simulink model "HvacActrPosnFilFrnt"  (R2022b)
settings: Solver=FixedStepDiscrete  StartTime=0.0  StopTime=10.0  FixedStep=0.2  RelTol=1e-3
settings Model: Creator=blue.ge  LastModifiedBy=blue.ge
settings DataIOCC: SaveFormat=StructureWithTime
settings OptimizationCC: DefaultParameterBehavior=Inlined  EnhancedBackFolding=on  PassReuseOutputArgsAs=Individual arguments  ZeroExternalMemoryAtStartup=off  ZeroInternalMemoryAtStartup=off  OptimizeBlockOrder=speed  DifferentSizesBufferReuse=on  OptimizationCustomize=off  EfficientTunableParamExpr=on
settings DebuggingCC: ParameterTunabilityLossMsg=error
settings ModelReferenceCC: ModelReferenceNumInstancesAllowed=Single
settings SFSimCC: MATLABDynamicMemAlloc=off
settings RTWCC: Description=AUTOSAR  SystemTargetFile=autosar.tlc  GenCodeOnly=on  TemplateMakefile=ert_default_tmf
settings CodeAppCC: ForceParamTrailComments=on  IgnoreCustomStorageClasses=off  OperatorAnnotations=on  SimulinkDataObjDesc=on  SFDataObjDesc=on  InsertBlockDesc=on
settings STFCustomTargetCC: TargetLangStandard=C99 (ISO)  MultiwordLength=256  ParMdlRefBuildCompliant=on  CompOptLevelCompliant=on  IncludeMdlTerminateFcn=off  CombineOutputUpdateFcns=on  SuppressErrorStatus=on  ERTCustomFileBanners=on  SupportNonFinite=off  SupportComplex=off  SupportContinuousTime=off  SupportNonInlinedSFcns=off  RemoveResetFunc=on  MATLABClassNameForMDSCustomization=Simulink.SoftwareTarget.ERTCustomization  AutosarCompliant=on  UseToolchainInfoCompliant=on  LookupTableObjectStructAxisOrder=2,1,3,4,...  LUTObjectStructOrderEvenSpacing=Size,Table,Breakpoints  SystemTargetFile=autosar.tlc
settings ERTTargetCC: TargetLangStandard=C99 (ISO)  ConcurrentExecutionCompliant=off  IncludeMdlTerminateFcn=off  SuppressErrorStatus=on  SupportNonFinite=off  SupportComplex=off  ModelStepFunctionPrototypeControlCompliant=off  CPPClassGenCompliant=off  AutosarCompliant=on  LookupTableObjectStructAxisOrder=2,1,3,4,...  LUTObjectStructOrderEvenSpacing=Size,Table,Breakpoints  InlinedParameterPlacement=NonHierarchical  ERTCustomFileTemplate=example_file_process.tlc

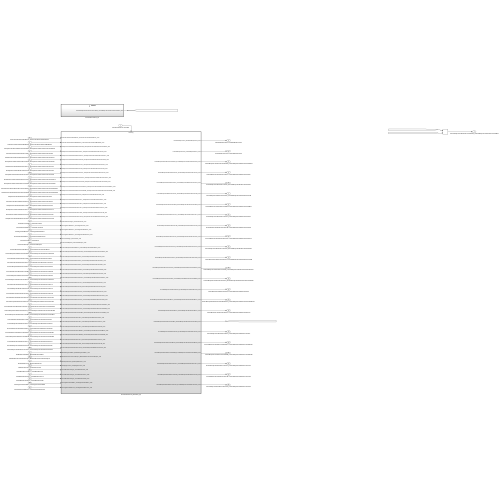
[thumbnail: Simulink's stored preview of the top-level diagram]
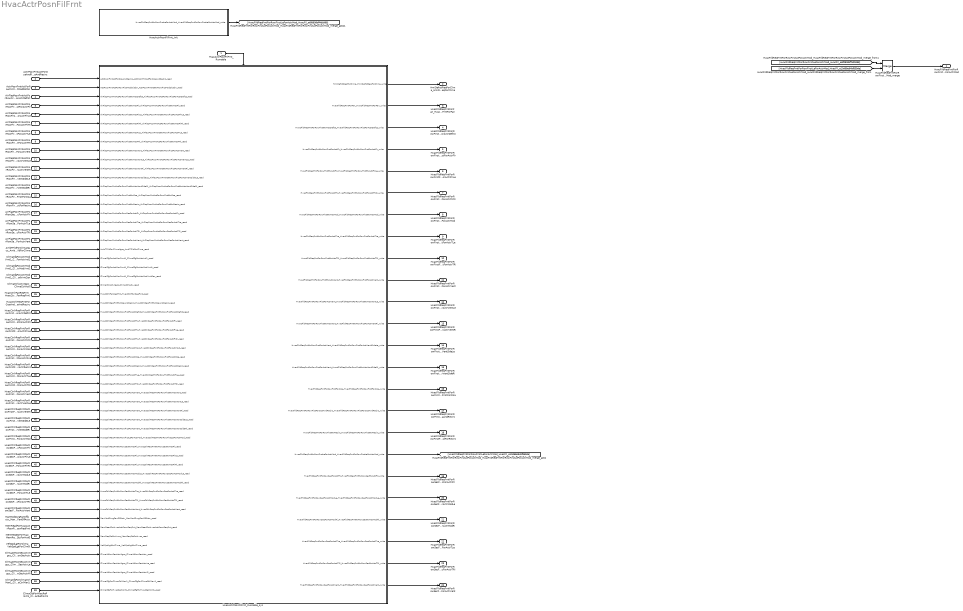
== system HvacActrPosnFilFrnt ==
  HvacActrPosnFilFrnt_Runnable : Inport  SampleTime=0.2  OutputFunctionCall=on
  ActrPosnFrntActForOsaAndRecirc_ActrPosnFrntActForOsaAndRecirc : Inport  Port=2  OutMin=0  OutMax=100  OutDataTypeStr=Perc9  PortDimensions=1
  ActrPosnFrntActForRowFirstModDistbn_ActrPosnFrntActForRowFirstModDistbn : Inport  Port=3  OutMin=0  OutMax=100  OutDataTypeStr=Perc9  PortDimensions=1
  AirFlapPosnFrntActForRowFirstForActrDefrst_AirFlapPosnFrntActForRowFirstForActrDefrst : Inport  Port=4  OutMin=0  OutMax=100  OutDataTypeStr=Perc9  PortDimensions=1
  AirFlapPosnFrntActForRowFirstForActrFlr_AirFlapPosnFrntActForRowFirstForActrFlr : Inport  Port=5  OutMin=0  OutMax=100  OutDataTypeStr=Perc9  PortDimensions=1
  AirFlapPosnFrntActForRowFirstForActrFlrLe_AirFlapPosnFrntActForRowFirstForActrFlrLe : Inport  Port=6  OutMin=0  OutMax=100  OutDataTypeStr=Perc9  PortDimensions=1
  AirFlapPosnFrntActForRowFirstForActrFlrRi_AirFlapPosnFrntActForRowFirstForActrFlrRi : Inport  Port=7  OutMin=0  OutMax=100  OutDataTypeStr=Perc9  PortDimensions=1
  AirFlapPosnFrntActForRowFirstForActrTLe_AirFlapPosnFrntActForRowFirstForActrTLe : Inport  Port=8  OutMin=0  OutMax=100  OutDataTypeStr=Perc9  PortDimensions=1
  AirFlapPosnFrntActForRowFirstForActrTRi_AirFlapPosnFrntActForRowFirstForActrTRi : Inport  Port=9  OutMin=0  OutMax=100  OutDataTypeStr=Perc9  PortDimensions=1
  AirFlapPosnFrntActForRowFirstForActrVent_AirFlapPosnFrntActForRowFirstForActrVent : Inport  Port=10  OutMin=0  OutMax=100  OutDataTypeStr=Perc9  PortDimensions=1
  AirFlapPosnFrntActForRowFirstForActrVentLe_AirFlapPosnFrntActForRowFirstForActrVentLe : Inport  Port=11  OutMin=0  OutMax=100  OutDataTypeStr=Perc9  PortDimensions=1
  AirFlapPosnFrntActForRowFirstForActrVentRi_AirFlapPosnFrntActForRowFirstForActrVentRi : Inport  Port=12  OutMin=0  OutMax=100  OutDataTypeStr=Perc9  PortDimensions=1
  AirFlapPosnFrntActForRowFirstForActrVentSideLe_AirFlapPosnFrntActForRowFirstForActrVentSideLe : Inport  Port=13  OutMin=0  OutMax=100  OutDataTypeStr=Perc9  PortDimensions=1
  AirFlapPosnFrntActForRowFirstForActrVentSideRi_AirFlapPosnFrntActForRowFirstForActrVentSideRi : Inport  Port=14  OutMin=0  OutMax=100  OutDataTypeStr=Perc9  PortDimensions=1
  AirFlapPosnFrntActForRowFirstForOsa_AirFlapPosnFrntActForRowFirstForOsa : Inport  Port=15  OutMin=0  OutMax=100  OutDataTypeStr=Perc9  PortDimensions=1
  AirFlapPosnFrntActForRowFirstForRecirc_AirFlapPosnFrntActForRowFirstForRecirc : Inport  Port=16  OutMin=0  OutMax=100  OutDataTypeStr=Perc9  PortDimensions=1
  AirFlapPosnFrntActForRowSecForActrFlr_AirFlapPosnFrntActForRowSecForActrFlr : Inport  Port=17  OutMin=0  OutMax=100  OutDataTypeStr=Perc9  PortDimensions=1
  AirFlapPosnFrntActForRowSecForActrTLe_AirFlapPosnFrntActForRowSecForActrTLe : Inport  Port=18  OutMin=0  OutMax=100  OutDataTypeStr=Perc9  PortDimensions=1
  AirFlapPosnFrntActForRowSecForActrTRi_AirFlapPosnFrntActForRowSecForActrTRi : Inport  Port=19  OutMin=0  OutMax=100  OutDataTypeStr=Perc9  PortDimensions=1
  AirFlapPosnFrntActForRowSecForActrVent_AirFlapPosnFrntActForRowSecForActrVent : Inport  Port=20  OutMin=0  OutMax=100  OutDataTypeStr=Perc9  PortDimensions=1
  AmbTFildForClimaNgcc_AmbTFildForClima : Inport  Port=21  OutDataTypeStr=T30  PortDimensions=1
  ClimaCfgForActrModVrnt1_ClimaCfgForActrInd1 : Inport  Port=22  OutDataTypeStr=Enum: ClimaCfgForActrInd1  PortDimensions=1
  ClimaCfgForActrModVrnt1_ClimaCfgForActrModVrnt1 : Inport  Port=23  OutDataTypeStr=Enum: ClimaCfgForActrModVrnt1  PortDimensions=1
  ClimaCfgForActrModVrnt1_ClimaCfgForActrModVrntCen : Inport  Port=24  OutDataTypeStr=Enum: ClimaCfgForActrModVrntCen  PortDimensions=1
  ClimaCtrlActvNgcc_ClimaCtrlActv : Inport  Port=25  OutDataTypeStr=Boolean  PortDimensions=1
  HvacCtrlrFanReqFrnt_HvacCtrlrFanReqFrnt : Inport  Port=26  OutDataTypeStr=FanSpd1  PortDimensions=1
  HvacCtrlrReqFrntForOsaAndRecirc_HvacCtrlrReqFrntForOsaAndRecirc : Inport  Port=27  OutDataTypeStr=Perc30  PortDimensions=1
  HvacCtrlrReqFrntForRowFirstForActrDefrst_HvacCtrlrReqFrntForRowFirstForActrDefrst : Inport  Port=28  OutDataTypeStr=Perc30  PortDimensions=1
  HvacCtrlrReqFrntForRowFirstForActrFlr_HvacCtrlrReqFrntForRowFirstForActrFlr : Inport  Port=29  OutDataTypeStr=Perc30  PortDimensions=1
  HvacCtrlrReqFrntForRowFirstForActrFlr_HvacCtrlrReqFrntForRowFirstForActrFlrLe : Inport  Port=30  OutDataTypeStr=Perc30  PortDimensions=1
  HvacCtrlrReqFrntForRowFirstForActrFlr_HvacCtrlrReqFrntForRowFirstForActrFlrRi : Inport  Port=31  OutDataTypeStr=Perc30  PortDimensions=1
  HvacCtrlrReqFrntForRowFirstForActrMod_HvacCtrlrReqFrntForRowFirstForActrMod : Inport  Port=32  OutDataTypeStr=Perc30  PortDimensions=1
  HvacCtrlrReqFrntForRowFirstForActrOsa_HvacCtrlrReqFrntForRowFirstForActrOsa : Inport  Port=33  OutDataTypeStr=Perc30  PortDimensions=1
  HvacCtrlrReqFrntForRowFirstForActrRecirc_HvacCtrlrReqFrntForRowFirstForActrRecirc : Inport  Port=34  OutDataTypeStr=Perc30  PortDimensions=1
  HvacCtrlrReqFrntForRowFirstForActrTLe_HvacCtrlrReqFrntForRowFirstForActrTLe : Inport  Port=35  OutDataTypeStr=Perc30  PortDimensions=1
  HvacCtrlrReqFrntForRowFirstForActrTRi_HvacCtrlrReqFrntForRowFirstForActrTRi : Inport  Port=36  OutDataTypeStr=Perc30  PortDimensions=1
  HvacCtrlrReqFrntForRowFirstForActrVent_HvacCtrlrReqFrntForRowFirstForActrVent : Inport  Port=37  OutDataTypeStr=Perc30  PortDimensions=1
  HvacCtrlrReqFrntForRowFirstForActrVent_HvacCtrlrReqFrntForRowFirstForActrVentLe : Inport  Port=38  OutDataTypeStr=Perc30  PortDimensions=1
  HvacCtrlrReqFrntForRowFirstForActrVent_HvacCtrlrReqFrntForRowFirstForActrVentRi : Inport  Port=39  OutDataTypeStr=Perc30  PortDimensions=1
  HvacCtrlrReqFrntForRowFirstForActrVent_HvacCtrlrReqFrntForRowFirstForActrVentSideLe : Inport  Port=40  OutDataTypeStr=Perc30  PortDimensions=1
  HvacCtrlrReqFrntForRowFirstForActrVent_HvacCtrlrReqFrntForRowFirstForActrVentSideRi : Inport  Port=41  OutDataTypeStr=Perc30  PortDimensions=1
  HvacCtrlrReqFrntForRowFirstLeForActrMod_HvacCtrlrReqFrntForRowFirstLeForActrMod : Inport  Port=42  OutDataTypeStr=Perc30  PortDimensions=1
  HvacCtrlrReqFrntForRowSecForActrFlr_HvacCtrlrReqFrntForRowSecForActrFlr : Inport  Port=43  OutDataTypeStr=Perc30  PortDimensions=1
  HvacCtrlrReqFrntForRowSecForActrFlr_HvacCtrlrReqFrntForRowSecForActrFlrLe : Inport  Port=44  OutDataTypeStr=Perc30  PortDimensions=1
  HvacCtrlrReqFrntForRowSecForActrFlr_HvacCtrlrReqFrntForRowSecForActrFlrRi : Inport  Port=45  OutDataTypeStr=Perc30  PortDimensions=1
  HvacCtrlrReqFrntForRowSecForActrModLe_HvacCtrlrReqFrntForRowSecForActrModLe : Inport  Port=46  OutDataTypeStr=Perc30  PortDimensions=1
  HvacCtrlrReqFrntForRowSecForActrModRi_HvacCtrlrReqFrntForRowSecForActrModRi : Inport  Port=47  OutDataTypeStr=Perc30  PortDimensions=1
  HvacCtrlrReqFrntForRowSecForActrTLe_HvacCtrlrReqFrntForRowSecForActrTLe : Inport  Port=48  OutDataTypeStr=Perc30  PortDimensions=1
  HvacCtrlrReqFrntForRowSecForActrTRi_HvacCtrlrReqFrntForRowSecForActrTRi : Inport  Port=49  OutDataTypeStr=Perc30  PortDimensions=1
  HvacCtrlrReqFrntForRowSecForActrVent_HvacCtrlrReqFrntForRowSecForActrVent : Inport  Port=50  OutDataTypeStr=Perc30  PortDimensions=1
  ManModDurgFanOffActv_ManModDurgFanOffActv : Inport  Port=51  OutDataTypeStr=Boolean  PortDimensions=1
  MemReadForHvacActrPosnReqFrnt_MemReadForHvacActrPosnReqFrnt : Inport  Port=52  OutDataTypeStr=Bus: MemRecForHvacActrPosnReqFrnt  PortDimensions=1  BusOutputAsStruct=on
  MemReqStsForHvac_MemReqStsForHvac : Inport  Port=53  OutDataTypeStr=Enum: MemReqSts  PortDimensions=1
  VehSpdLgtForClima_VehSpdLgtForClima : Inport  Port=54  OutDataTypeStr=Bus: VehSpdLgtForClima  PortDimensions=1  BusOutputAsStruct=on
  ClimaAtRowSecActvNgcc_ClimaAtRowSecActv : Inport  Port=55  OutDataTypeStr=Boolean  PortDimensions=1
  ClimaAtRowSecActvNgcc_ClimaAtRowSecActvLe : Inport  Port=56  OutDataTypeStr=Boolean  PortDimensions=1
  ClimaAtRowSecActvNgcc_ClimaAtRowSecActvRi : Inport  Port=57  OutDataTypeStr=Boolean  PortDimensions=1
  ClimaCfgForClimaCtrlMan1_ClimaCfgForClimaCtrlMan1 : Inport  Port=58  OutDataTypeStr=Boolean  PortDimensions=1
  ClimaCfgForHvacRePrsnt1_ClimaCfgForHvacRePrsnt1 : Inport  Port=59  OutDataTypeStr=Enum: CarCfgForHvacRe  PortDimensions=1
  HvacActrPosnFilFrnt_Init : Subsystem
  HvacActrPosnFilFrnt_Runnable_sys : Subsystem
  HvacFildReqFrntForRowFirstLeForActrMod_HvacFildReqFrntForRowFirstLeForActrMod_merge : Merge
  HvacFildReqFrntForRowFirstLeForActrMod_HvacFildReqFrntForRowFirstLeForActrMod : Outport  OutDataTypeStr=Perc30  PortDimensions=1
  HmiDefrstReqForClima_HmiDefrstReqForClima : Outport  Port=2  OutDataTypeStr=Enum: OnOff1  PortDimensions=1
  HvacFildReqFrntForFan_HvacFildReqFrntForFan : Outport  Port=3  OutDataTypeStr=HvacFanSpd  PortDimensions=1
  HvacFildReqFrntForRowFirstForActrDefrst_HvacFildReqFrntForRowFirstForActrDefrst : Outport  Port=4  OutDataTypeStr=Perc30  PortDimensions=1
  HvacFildReqFrntForRowFirstForActrFlr_HvacFildReqFrntForRowFirstForActrFlr : Outport  Port=5  OutDataTypeStr=Perc30  PortDimensions=1
  HvacFildReqFrntForRowFirstForActrFlr_HvacFildReqFrntForRowFirstForActrFlrLe : Outport  Port=6  OutDataTypeStr=Perc30  PortDimensions=1
  HvacFildReqFrntForRowFirstForActrFlr_HvacFildReqFrntForRowFirstForActrFlrRi : Outport  Port=7  OutDataTypeStr=Perc30  PortDimensions=1
  HvacFildReqFrntForRowFirstForActrMod_HvacFildReqFrntForRowFirstForActrMod : Outport  Port=8  OutDataTypeStr=Perc30  PortDimensions=1
  HvacFildReqFrntForRowFirstForActrTLe_HvacFildReqFrntForRowFirstForActrTLe : Outport  Port=9  OutDataTypeStr=Perc30  PortDimensions=1
  HvacFildReqFrntForRowFirstForActrTRi_HvacFildReqFrntForRowFirstForActrTRi : Outport  Port=10  OutDataTypeStr=Perc30  PortDimensions=1
  HvacFildReqFrntForRowFirstForActrVent_HvacFildReqFrntForRowFirstForActrVent : Outport  Port=11  OutDataTypeStr=Perc30  PortDimensions=1
  HvacFildReqFrntForRowFirstForActrVent_HvacFildReqFrntForRowFirstForActrVentLe : Outport  Port=12  OutDataTypeStr=Perc30  PortDimensions=1
  HvacFildReqFrntForRowFirstForActrVent_HvacFildReqFrntForRowFirstForActrVentRi : Outport  Port=13  OutDataTypeStr=Perc30  PortDimensions=1
  HvacFildReqFrntForRowFirstForActrVent_HvacFildReqFrntForRowFirstForActrVentSideLe : Outport  Port=14  OutDataTypeStr=Perc30  PortDimensions=1
  HvacFildReqFrntForRowFirstForActrVent_HvacFildReqFrntForRowFirstForActrVentSideRi : Outport  Port=15  OutDataTypeStr=Perc30  PortDimensions=1
  HvacFildReqFrntForRowFirstForOsa_HvacFildReqFrntForRowFirstForOsa : Outport  Port=16  OutDataTypeStr=Perc30  PortDimensions=1
  HvacFildReqFrntForRowFirstForOsaAndRecirc_HvacFildReqFrntForRowFirstForOsaAndRecirc : Outport  Port=17  OutDataTypeStr=Perc30  PortDimensions=1
  HvacFildReqFrntForRowFirstForRecirc_HvacFildReqFrntForRowFirstForRecirc : Outport  Port=18  OutDataTypeStr=Perc30  PortDimensions=1
  HvacFildReqFrntForRowSecForActrFlr_HvacFildReqFrntForRowSecForActrFlr : Outport  Port=19  OutDataTypeStr=Perc30  PortDimensions=1
  HvacFildReqFrntForRowSecForActrModLe_HvacFildReqFrntForRowSecForActrModLe : Outport  Port=20  OutDataTypeStr=Perc30  PortDimensions=1
  HvacFildReqFrntForRowSecForActrModRi_HvacFildReqFrntForRowSecForActrModRi : Outport  Port=21  OutDataTypeStr=Perc30  PortDimensions=1
  HvacFildReqFrntForRowSecForActrTLe_HvacFildReqFrntForRowSecForActrTLe : Outport  Port=22  OutDataTypeStr=Perc30  PortDimensions=1
  HvacFildReqFrntForRowSecForActrTRi_HvacFildReqFrntForRowSecForActrTRi : Outport  Port=23  OutDataTypeStr=Perc30  PortDimensions=1
  HvacFildReqFrntForRowSecForActrVent_HvacFildReqFrntForRowSecForActrVent : Outport  Port=24  OutDataTypeStr=Perc30  PortDimensions=1
  wires:
  ActrPosnFrntActForOsaAndRecirc_ActrPosnFrntActForOsaAndRecirc/1 -> HvacActrPosnFilFrnt_Runnable_sys/1(ActrPosnFrntActForOsaAndRecirc_ActrPosnFrntActForOsaAndRecirc_read)
  ActrPosnFrntActForRowFirstModDistbn_ActrPosnFrntActForRowFirstModDistbn/1 -> HvacActrPosnFilFrnt_Runnable_sys/2(ActrPosnFrntActForRowFirstModDistbn_ActrPosnFrntActForRowFirstModDistbn_read)
  AirFlapPosnFrntActForRowFirstForActrDefrst_AirFlapPosnFrntActForRowFirstForActrDefrst/1 -> HvacActrPosnFilFrnt_Runnable_sys/3(AirFlapPosnFrntActForRowFirstForActrDefrst_AirFlapPosnFrntActForRowFirstForActrDefrst_read)
  AirFlapPosnFrntActForRowFirstForActrFlr_AirFlapPosnFrntActForRowFirstForActrFlr/1 -> HvacActrPosnFilFrnt_Runnable_sys/4(AirFlapPosnFrntActForRowFirstForActrFlr_AirFlapPosnFrntActForRowFirstForActrFlr_read)
  AirFlapPosnFrntActForRowFirstForActrFlrLe_AirFlapPosnFrntActForRowFirstForActrFlrLe/1 -> HvacActrPosnFilFrnt_Runnable_sys/5(AirFlapPosnFrntActForRowFirstForActrFlrLe_AirFlapPosnFrntActForRowFirstForActrFlrLe_read)
  AirFlapPosnFrntActForRowFirstForActrFlrRi_AirFlapPosnFrntActForRowFirstForActrFlrRi/1 -> HvacActrPosnFilFrnt_Runnable_sys/6(AirFlapPosnFrntActForRowFirstForActrFlrRi_AirFlapPosnFrntActForRowFirstForActrFlrRi_read)
  AirFlapPosnFrntActForRowFirstForActrTLe_AirFlapPosnFrntActForRowFirstForActrTLe/1 -> HvacActrPosnFilFrnt_Runnable_sys/7(AirFlapPosnFrntActForRowFirstForActrTLe_AirFlapPosnFrntActForRowFirstForActrTLe_read)
  AirFlapPosnFrntActForRowFirstForActrTRi_AirFlapPosnFrntActForRowFirstForActrTRi/1 -> HvacActrPosnFilFrnt_Runnable_sys/8(AirFlapPosnFrntActForRowFirstForActrTRi_AirFlapPosnFrntActForRowFirstForActrTRi_read)
  AirFlapPosnFrntActForRowFirstForActrVent_AirFlapPosnFrntActForRowFirstForActrVent/1 -> HvacActrPosnFilFrnt_Runnable_sys/9(AirFlapPosnFrntActForRowFirstForActrVent_AirFlapPosnFrntActForRowFirstForActrVent_read)
  AirFlapPosnFrntActForRowFirstForActrVentLe_AirFlapPosnFrntActForRowFirstForActrVentLe/1 -> HvacActrPosnFilFrnt_Runnable_sys/10(AirFlapPosnFrntActForRowFirstForActrVentLe_AirFlapPosnFrntActForRowFirstForActrVentLe_read)
  AirFlapPosnFrntActForRowFirstForActrVentRi_AirFlapPosnFrntActForRowFirstForActrVentRi/1 -> HvacActrPosnFilFrnt_Runnable_sys/11(AirFlapPosnFrntActForRowFirstForActrVentRi_AirFlapPosnFrntActForRowFirstForActrVentRi_read)
  AirFlapPosnFrntActForRowFirstForActrVentSideLe_AirFlapPosnFrntActForRowFirstForActrVentSideLe/1 -> HvacActrPosnFilFrnt_Runnable_sys/12(AirFlapPosnFrntActForRowFirstForActrVentSideLe_AirFlapPosnFrntActForRowFirstForActrVentSideLe_read)
  AirFlapPosnFrntActForRowFirstForActrVentSideRi_AirFlapPosnFrntActForRowFirstForActrVentSideRi/1 -> HvacActrPosnFilFrnt_Runnable_sys/13(AirFlapPosnFrntActForRowFirstForActrVentSideRi_AirFlapPosnFrntActForRowFirstForActrVentSideRi_read)
  AirFlapPosnFrntActForRowFirstForOsa_AirFlapPosnFrntActForRowFirstForOsa/1 -> HvacActrPosnFilFrnt_Runnable_sys/14(AirFlapPosnFrntActForRowFirstForOsa_AirFlapPosnFrntActForRowFirstForOsa_read)
  AirFlapPosnFrntActForRowFirstForRecirc_AirFlapPosnFrntActForRowFirstForRecirc/1 -> HvacActrPosnFilFrnt_Runnable_sys/15(AirFlapPosnFrntActForRowFirstForRecirc_AirFlapPosnFrntActForRowFirstForRecirc_read)
  AirFlapPosnFrntActForRowSecForActrFlr_AirFlapPosnFrntActForRowSecForActrFlr/1 -> HvacActrPosnFilFrnt_Runnable_sys/16(AirFlapPosnFrntActForRowSecForActrFlr_AirFlapPosnFrntActForRowSecForActrFlr_read)
  AirFlapPosnFrntActForRowSecForActrTLe_AirFlapPosnFrntActForRowSecForActrTLe/1 -> HvacActrPosnFilFrnt_Runnable_sys/17(AirFlapPosnFrntActForRowSecForActrTLe_AirFlapPosnFrntActForRowSecForActrTLe_read)
  AirFlapPosnFrntActForRowSecForActrTRi_AirFlapPosnFrntActForRowSecForActrTRi/1 -> HvacActrPosnFilFrnt_Runnable_sys/18(AirFlapPosnFrntActForRowSecForActrTRi_AirFlapPosnFrntActForRowSecForActrTRi_read)
  AirFlapPosnFrntActForRowSecForActrVent_AirFlapPosnFrntActForRowSecForActrVent/1 -> HvacActrPosnFilFrnt_Runnable_sys/19(AirFlapPosnFrntActForRowSecForActrVent_AirFlapPosnFrntActForRowSecForActrVent_read)
  AmbTFildForClimaNgcc_AmbTFildForClima/1 -> HvacActrPosnFilFrnt_Runnable_sys/20(AmbTFildForClimaNgcc_AmbTFildForClima_read)
  ClimaCfgForActrModVrnt1_ClimaCfgForActrInd1/1 -> HvacActrPosnFilFrnt_Runnable_sys/21(ClimaCfgForActrModVrnt1_ClimaCfgForActrInd1_read)
  ClimaCfgForActrModVrnt1_ClimaCfgForActrModVrnt1/1 -> HvacActrPosnFilFrnt_Runnable_sys/22(ClimaCfgForActrModVrnt1_ClimaCfgForActrModVrnt1_read)
  ClimaCfgForActrModVrnt1_ClimaCfgForActrModVrntCen/1 -> HvacActrPosnFilFrnt_Runnable_sys/23(ClimaCfgForActrModVrnt1_ClimaCfgForActrModVrntCen_read)
  ClimaCtrlActvNgcc_ClimaCtrlActv/1 -> HvacActrPosnFilFrnt_Runnable_sys/24(ClimaCtrlActvNgcc_ClimaCtrlActv_read)
  HvacCtrlrFanReqFrnt_HvacCtrlrFanReqFrnt/1 -> HvacActrPosnFilFrnt_Runnable_sys/25(HvacCtrlrFanReqFrnt_HvacCtrlrFanReqFrnt_read)
  HvacCtrlrReqFrntForOsaAndRecirc_HvacCtrlrReqFrntForOsaAndRecirc/1 -> HvacActrPosnFilFrnt_Runnable_sys/26(HvacCtrlrReqFrntForOsaAndRecirc_HvacCtrlrReqFrntForOsaAndRecirc_read)
  HvacCtrlrReqFrntForRowFirstForActrDefrst_HvacCtrlrReqFrntForRowFirstForActrDefrst/1 -> HvacActrPosnFilFrnt_Runnable_sys/27(HvacCtrlrReqFrntForRowFirstForActrDefrst_HvacCtrlrReqFrntForRowFirstForActrDefrst_read)
  HvacCtrlrReqFrntForRowFirstForActrFlr_HvacCtrlrReqFrntForRowFirstForActrFlr/1 -> HvacActrPosnFilFrnt_Runnable_sys/28(HvacCtrlrReqFrntForRowFirstForActrFlr_HvacCtrlrReqFrntForRowFirstForActrFlr_read)
  HvacCtrlrReqFrntForRowFirstForActrFlr_HvacCtrlrReqFrntForRowFirstForActrFlrLe/1 -> HvacActrPosnFilFrnt_Runnable_sys/29(HvacCtrlrReqFrntForRowFirstForActrFlr_HvacCtrlrReqFrntForRowFirstForActrFlrLe_read)
  HvacCtrlrReqFrntForRowFirstForActrFlr_HvacCtrlrReqFrntForRowFirstForActrFlrRi/1 -> HvacActrPosnFilFrnt_Runnable_sys/30(HvacCtrlrReqFrntForRowFirstForActrFlr_HvacCtrlrReqFrntForRowFirstForActrFlrRi_read)
  HvacCtrlrReqFrntForRowFirstForActrMod_HvacCtrlrReqFrntForRowFirstForActrMod/1 -> HvacActrPosnFilFrnt_Runnable_sys/31(HvacCtrlrReqFrntForRowFirstForActrMod_HvacCtrlrReqFrntForRowFirstForActrMod_read)
  HvacCtrlrReqFrntForRowFirstForActrOsa_HvacCtrlrReqFrntForRowFirstForActrOsa/1 -> HvacActrPosnFilFrnt_Runnable_sys/32(HvacCtrlrReqFrntForRowFirstForActrOsa_HvacCtrlrReqFrntForRowFirstForActrOsa_read)
  HvacCtrlrReqFrntForRowFirstForActrRecirc_HvacCtrlrReqFrntForRowFirstForActrRecirc/1 -> HvacActrPosnFilFrnt_Runnable_sys/33(HvacCtrlrReqFrntForRowFirstForActrRecirc_HvacCtrlrReqFrntForRowFirstForActrRecirc_read)
  HvacCtrlrReqFrntForRowFirstForActrTLe_HvacCtrlrReqFrntForRowFirstForActrTLe/1 -> HvacActrPosnFilFrnt_Runnable_sys/34(HvacCtrlrReqFrntForRowFirstForActrTLe_HvacCtrlrReqFrntForRowFirstForActrTLe_read)
  HvacCtrlrReqFrntForRowFirstForActrTRi_HvacCtrlrReqFrntForRowFirstForActrTRi/1 -> HvacActrPosnFilFrnt_Runnable_sys/35(HvacCtrlrReqFrntForRowFirstForActrTRi_HvacCtrlrReqFrntForRowFirstForActrTRi_read)
  HvacCtrlrReqFrntForRowFirstForActrVent_HvacCtrlrReqFrntForRowFirstForActrVent/1 -> HvacActrPosnFilFrnt_Runnable_sys/36(HvacCtrlrReqFrntForRowFirstForActrVent_HvacCtrlrReqFrntForRowFirstForActrVent_read)
  HvacCtrlrReqFrntForRowFirstForActrVent_HvacCtrlrReqFrntForRowFirstForActrVentLe/1 -> HvacActrPosnFilFrnt_Runnable_sys/37(HvacCtrlrReqFrntForRowFirstForActrVent_HvacCtrlrReqFrntForRowFirstForActrVentLe_read)
  HvacCtrlrReqFrntForRowFirstForActrVent_HvacCtrlrReqFrntForRowFirstForActrVentRi/1 -> HvacActrPosnFilFrnt_Runnable_sys/38(HvacCtrlrReqFrntForRowFirstForActrVent_HvacCtrlrReqFrntForRowFirstForActrVentRi_read)
  HvacCtrlrReqFrntForRowFirstForActrVent_HvacCtrlrReqFrntForRowFirstForActrVentSideLe/1 -> HvacActrPosnFilFrnt_Runnable_sys/39(HvacCtrlrReqFrntForRowFirstForActrVent_HvacCtrlrReqFrntForRowFirstForActrVentSideLe_read)
  HvacCtrlrReqFrntForRowFirstForActrVent_HvacCtrlrReqFrntForRowFirstForActrVentSideRi/1 -> HvacActrPosnFilFrnt_Runnable_sys/40(HvacCtrlrReqFrntForRowFirstForActrVent_HvacCtrlrReqFrntForRowFirstForActrVentSideRi_read)
  HvacCtrlrReqFrntForRowFirstLeForActrMod_HvacCtrlrReqFrntForRowFirstLeForActrMod/1 -> HvacActrPosnFilFrnt_Runnable_sys/41(HvacCtrlrReqFrntForRowFirstLeForActrMod_HvacCtrlrReqFrntForRowFirstLeForActrMod_read)
  HvacCtrlrReqFrntForRowSecForActrFlr_HvacCtrlrReqFrntForRowSecForActrFlr/1 -> HvacActrPosnFilFrnt_Runnable_sys/42(HvacCtrlrReqFrntForRowSecForActrFlr_HvacCtrlrReqFrntForRowSecForActrFlr_read)
  HvacCtrlrReqFrntForRowSecForActrFlr_HvacCtrlrReqFrntForRowSecForActrFlrLe/1 -> HvacActrPosnFilFrnt_Runnable_sys/43(HvacCtrlrReqFrntForRowSecForActrFlr_HvacCtrlrReqFrntForRowSecForActrFlrLe_read)
  HvacCtrlrReqFrntForRowSecForActrFlr_HvacCtrlrReqFrntForRowSecForActrFlrRi/1 -> HvacActrPosnFilFrnt_Runnable_sys/44(HvacCtrlrReqFrntForRowSecForActrFlr_HvacCtrlrReqFrntForRowSecForActrFlrRi_read)
  HvacCtrlrReqFrntForRowSecForActrModLe_HvacCtrlrReqFrntForRowSecForActrModLe/1 -> HvacActrPosnFilFrnt_Runnable_sys/45(HvacCtrlrReqFrntForRowSecForActrModLe_HvacCtrlrReqFrntForRowSecForActrModLe_read)
  HvacCtrlrReqFrntForRowSecForActrModRi_HvacCtrlrReqFrntForRowSecForActrModRi/1 -> HvacActrPosnFilFrnt_Runnable_sys/46(HvacCtrlrReqFrntForRowSecForActrModRi_HvacCtrlrReqFrntForRowSecForActrModRi_read)
  HvacCtrlrReqFrntForRowSecForActrTLe_HvacCtrlrReqFrntForRowSecForActrTLe/1 -> HvacActrPosnFilFrnt_Runnable_sys/47(HvacCtrlrReqFrntForRowSecForActrTLe_HvacCtrlrReqFrntForRowSecForActrTLe_read)
  HvacCtrlrReqFrntForRowSecForActrTRi_HvacCtrlrReqFrntForRowSecForActrTRi/1 -> HvacActrPosnFilFrnt_Runnable_sys/48(HvacCtrlrReqFrntForRowSecForActrTRi_HvacCtrlrReqFrntForRowSecForActrTRi_read)
  HvacCtrlrReqFrntForRowSecForActrVent_HvacCtrlrReqFrntForRowSecForActrVent/1 -> HvacActrPosnFilFrnt_Runnable_sys/49(HvacCtrlrReqFrntForRowSecForActrVent_HvacCtrlrReqFrntForRowSecForActrVent_read)
  ManModDurgFanOffActv_ManModDurgFanOffActv/1 -> HvacActrPosnFilFrnt_Runnable_sys/50(ManModDurgFanOffActv_ManModDurgFanOffActv_read)
  MemReadForHvacActrPosnReqFrnt_MemReadForHvacActrPosnReqFrnt/1 -> HvacActrPosnFilFrnt_Runnable_sys/51(MemReadForHvacActrPosnReqFrnt_MemReadForHvacActrPosnReqFrnt_read)
  MemReqStsForHvac_MemReqStsForHvac/1 -> HvacActrPosnFilFrnt_Runnable_sys/52(MemReqStsForHvac_MemReqStsForHvac_read)
  VehSpdLgtForClima_VehSpdLgtForClima/1 -> HvacActrPosnFilFrnt_Runnable_sys/53(VehSpdLgtForClima_VehSpdLgtForClima_read)
  ClimaAtRowSecActvNgcc_ClimaAtRowSecActv/1 -> HvacActrPosnFilFrnt_Runnable_sys/54(ClimaAtRowSecActvNgcc_ClimaAtRowSecActv_read)
  ClimaAtRowSecActvNgcc_ClimaAtRowSecActvLe/1 -> HvacActrPosnFilFrnt_Runnable_sys/55(ClimaAtRowSecActvNgcc_ClimaAtRowSecActvLe_read)
  ClimaAtRowSecActvNgcc_ClimaAtRowSecActvRi/1 -> HvacActrPosnFilFrnt_Runnable_sys/56(ClimaAtRowSecActvNgcc_ClimaAtRowSecActvRi_read)
  ClimaCfgForClimaCtrlMan1_ClimaCfgForClimaCtrlMan1/1 -> HvacActrPosnFilFrnt_Runnable_sys/57(ClimaCfgForClimaCtrlMan1_ClimaCfgForClimaCtrlMan1_read)
  ClimaCfgForHvacRePrsnt1_ClimaCfgForHvacRePrsnt1/1 -> HvacActrPosnFilFrnt_Runnable_sys/58(ClimaCfgForHvacRePrsnt1_ClimaCfgForHvacRePrsnt1_read)
  HvacActrPosnFilFrnt_Runnable_sys/1(HmiDefrstReqForClima_HmiDefrstReqForClima_write) -> HmiDefrstReqForClima_HmiDefrstReqForClima/1
  HvacActrPosnFilFrnt_Runnable_sys/2(HvacFildReqFrntForFan_HvacFildReqFrntForFan_write) -> HvacFildReqFrntForFan_HvacFildReqFrntForFan/1
  HvacActrPosnFilFrnt_Runnable_sys/3(HvacFildReqFrntForRowFirstForActrDefrst_HvacFildReqFrntForRowFirstForActrDefrst_write) -> HvacFildReqFrntForRowFirstForActrDefrst_HvacFildReqFrntForRowFirstForActrDefrst/1
  HvacActrPosnFilFrnt_Runnable_sys/4(HvacFildReqFrntForRowFirstForActrFlr_HvacFildReqFrntForRowFirstForActrFlr_write) -> HvacFildReqFrntForRowFirstForActrFlr_HvacFildReqFrntForRowFirstForActrFlr/1
  HvacActrPosnFilFrnt_Runnable_sys/5(HvacFildReqFrntForRowFirstForActrFlr_HvacFildReqFrntForRowFirstForActrFlrLe_write) -> HvacFildReqFrntForRowFirstForActrFlr_HvacFildReqFrntForRowFirstForActrFlrLe/1
  HvacActrPosnFilFrnt_Runnable_sys/6(HvacFildReqFrntForRowFirstForActrFlr_HvacFildReqFrntForRowFirstForActrFlrRi_write) -> HvacFildReqFrntForRowFirstForActrFlr_HvacFildReqFrntForRowFirstForActrFlrRi/1
  HvacActrPosnFilFrnt_Runnable_sys/7(HvacFildReqFrntForRowFirstForActrMod_HvacFildReqFrntForRowFirstForActrMod_write) -> HvacFildReqFrntForRowFirstForActrMod_HvacFildReqFrntForRowFirstForActrMod/1
  HvacActrPosnFilFrnt_Runnable_sys/8(HvacFildReqFrntForRowFirstForActrTLe_HvacFildReqFrntForRowFirstForActrTLe_write) -> HvacFildReqFrntForRowFirstForActrTLe_HvacFildReqFrntForRowFirstForActrTLe/1
  HvacActrPosnFilFrnt_Runnable_sys/9(HvacFildReqFrntForRowFirstForActrTRi_HvacFildReqFrntForRowFirstForActrTRi_write) -> HvacFildReqFrntForRowFirstForActrTRi_HvacFildReqFrntForRowFirstForActrTRi/1
  HvacActrPosnFilFrnt_Runnable_sys/10(HvacFildReqFrntForRowFirstForActrVent_HvacFildReqFrntForRowFirstForActrVent_write) -> HvacFildReqFrntForRowFirstForActrVent_HvacFildReqFrntForRowFirstForActrVent/1
  HvacActrPosnFilFrnt_Runnable_sys/11(HvacFildReqFrntForRowFirstForActrVent_HvacFildReqFrntForRowFirstForActrVentLe_write) -> HvacFildReqFrntForRowFirstForActrVent_HvacFildReqFrntForRowFirstForActrVentLe/1
  HvacActrPosnFilFrnt_Runnable_sys/12(HvacFildReqFrntForRowFirstForActrVent_HvacFildReqFrntForRowFirstForActrVentRi_write) -> HvacFildReqFrntForRowFirstForActrVent_HvacFildReqFrntForRowFirstForActrVentRi/1
  HvacActrPosnFilFrnt_Runnable_sys/13(HvacFildReqFrntForRowFirstForActrVent_HvacFildReqFrntForRowFirstForActrVentSideLe_write) -> HvacFildReqFrntForRowFirstForActrVent_HvacFildReqFrntForRowFirstForActrVentSideLe/1
  HvacActrPosnFilFrnt_Runnable_sys/14(HvacFildReqFrntForRowFirstForActrVent_HvacFildReqFrntForRowFirstForActrVentSideRi_write) -> HvacFildReqFrntForRowFirstForActrVent_HvacFildReqFrntForRowFirstForActrVentSideRi/1
  HvacActrPosnFilFrnt_Runnable_sys/15(HvacFildReqFrntForRowFirstForOsa_HvacFildReqFrntForRowFirstForOsa_write) -> HvacFildReqFrntForRowFirstForOsa_HvacFildReqFrntForRowFirstForOsa/1
  HvacActrPosnFilFrnt_Runnable_sys/16(HvacFildReqFrntForRowFirstForOsaAndRecirc_HvacFildReqFrntForRowFirstForOsaAndRecirc_write) -> HvacFildReqFrntForRowFirstForOsaAndRecirc_HvacFildReqFrntForRowFirstForOsaAndRecirc/1
  HvacActrPosnFilFrnt_Runnable_sys/17(HvacFildReqFrntForRowFirstForRecirc_HvacFildReqFrntForRowFirstForRecirc_write) -> HvacFildReqFrntForRowFirstForRecirc_HvacFildReqFrntForRowFirstForRecirc/1
  HvacFildReqFrntForRowFirstLeForActrMod_HvacFildReqFrntForRowFirstLeForActrMod_merge/1 -> HvacFildReqFrntForRowFirstLeForActrMod_HvacFildReqFrntForRowFirstLeForActrMod/1
  HvacActrPosnFilFrnt_Runnable_sys/19(HvacFildReqFrntForRowSecForActrFlr_HvacFildReqFrntForRowSecForActrFlr_write) -> HvacFildReqFrntForRowSecForActrFlr_HvacFildReqFrntForRowSecForActrFlr/1
  HvacActrPosnFilFrnt_Runnable_sys/20(HvacFildReqFrntForRowSecForActrModLe_HvacFildReqFrntForRowSecForActrModLe_write) -> HvacFildReqFrntForRowSecForActrModLe_HvacFildReqFrntForRowSecForActrModLe/1
  HvacActrPosnFilFrnt_Runnable_sys/21(HvacFildReqFrntForRowSecForActrModRi_HvacFildReqFrntForRowSecForActrModRi_write) -> HvacFildReqFrntForRowSecForActrModRi_HvacFildReqFrntForRowSecForActrModRi/1
  HvacActrPosnFilFrnt_Runnable_sys/22(HvacFildReqFrntForRowSecForActrTLe_HvacFildReqFrntForRowSecForActrTLe_write) -> HvacFildReqFrntForRowSecForActrTLe_HvacFildReqFrntForRowSecForActrTLe/1
  HvacActrPosnFilFrnt_Runnable_sys/23(HvacFildReqFrntForRowSecForActrTRi_HvacFildReqFrntForRowSecForActrTRi_write) -> HvacFildReqFrntForRowSecForActrTRi_HvacFildReqFrntForRowSecForActrTRi/1
  HvacActrPosnFilFrnt_Runnable_sys/24(HvacFildReqFrntForRowSecForActrVent_HvacFildReqFrntForRowSecForActrVent_write) -> HvacFildReqFrntForRowSecForActrVent_HvacFildReqFrntForRowSecForActrVent/1
  HvacActrPosnFilFrnt_Runnable_sys/18(HvacFildReqFrntForRowFirstLeForActrMod_HvacFildReqFrntForRowFirstLeForActrMod_write) -> [HvacFildReqFrntForRowFirstLeForActrMod_HvacFil_e10d68e94bf859de]
  [HvacFildReqFrntForRowFirstLeForActrMod_HvacFil_e10d68e94bf859de] -> HvacFildReqFrntForRowFirstLeForActrMod_HvacFildReqFrntForRowFirstLeForActrMod_merge/2
  HvacActrPosnFilFrnt_Init/1(HvacFildReqFrntForRowFirstLeForActrMod_HvacFildReqFrntForRowFirstLeForActrMod_write) -> [HvacFildReqFrntForRowFirstLeForActrMod_HvacFil_e6fd808bff4db998]
  [HvacFildReqFrntForRowFirstLeForActrMod_HvacFil_e6fd808bff4db998] -> HvacFildReqFrntForRowFirstLeForActrMod_HvacFildReqFrntForRowFirstLeForActrMod_merge/1
  HvacActrPosnFilFrnt_Runnable/1 -> HvacActrPosnFilFrnt_Runnable_sys/Trigger

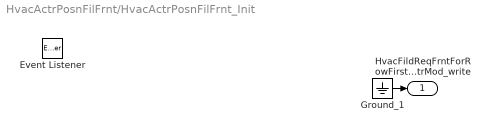
== system HvacActrPosnFilFrnt/HvacActrPosnFilFrnt_Init ==
  Event Listener : EventListener  EventName=reset
  Ground_1 : Ground
  HvacFildReqFrntForRowFirstLeForActrMod_HvacFildReqFrntForRowFirstLeForActrMod_write : Outport
  wires:
  Ground_1/1 -> HvacFildReqFrntForRowFirstLeForActrMod_HvacFildReqFrntForRowFirstLeForActrMod_write/1

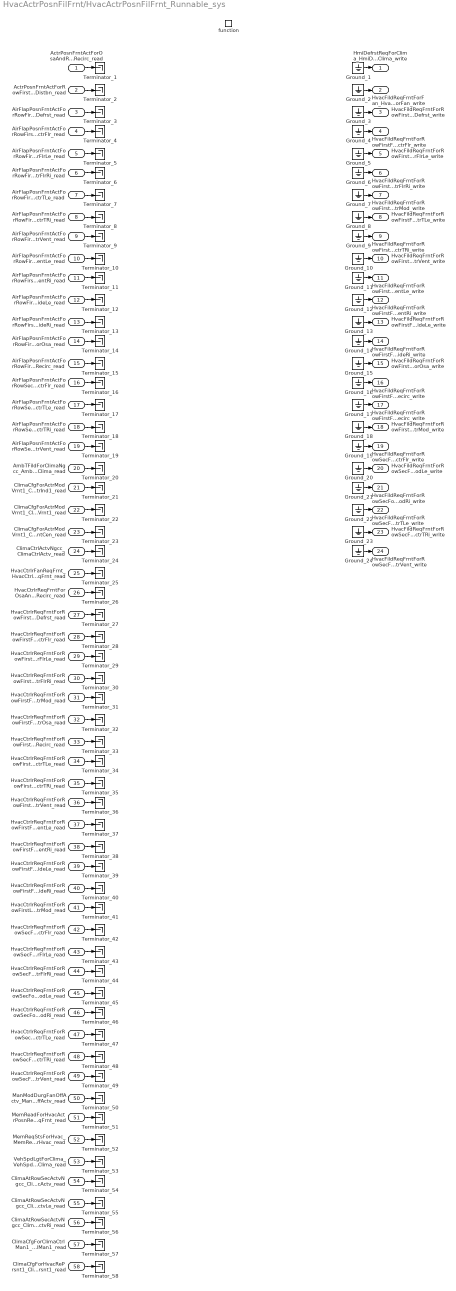
== system HvacActrPosnFilFrnt/HvacActrPosnFilFrnt_Runnable_sys ==
  ActrPosnFrntActForOsaAndRecirc_ActrPosnFrntActForOsaAndRecirc_read : Inport
  ActrPosnFrntActForRowFirstModDistbn_ActrPosnFrntActForRowFirstModDistbn_read : Inport  Port=2
  AirFlapPosnFrntActForRowFirstForActrDefrst_AirFlapPosnFrntActForRowFirstForActrDefrst_read : Inport  Port=3
  AirFlapPosnFrntActForRowFirstForActrFlr_AirFlapPosnFrntActForRowFirstForActrFlr_read : Inport  Port=4
  AirFlapPosnFrntActForRowFirstForActrFlrLe_AirFlapPosnFrntActForRowFirstForActrFlrLe_read : Inport  Port=5
  AirFlapPosnFrntActForRowFirstForActrFlrRi_AirFlapPosnFrntActForRowFirstForActrFlrRi_read : Inport  Port=6
  AirFlapPosnFrntActForRowFirstForActrTLe_AirFlapPosnFrntActForRowFirstForActrTLe_read : Inport  Port=7
  AirFlapPosnFrntActForRowFirstForActrTRi_AirFlapPosnFrntActForRowFirstForActrTRi_read : Inport  Port=8
  AirFlapPosnFrntActForRowFirstForActrVent_AirFlapPosnFrntActForRowFirstForActrVent_read : Inport  Port=9
  AirFlapPosnFrntActForRowFirstForActrVentLe_AirFlapPosnFrntActForRowFirstForActrVentLe_read : Inport  Port=10
  AirFlapPosnFrntActForRowFirstForActrVentRi_AirFlapPosnFrntActForRowFirstForActrVentRi_read : Inport  Port=11
  AirFlapPosnFrntActForRowFirstForActrVentSideLe_AirFlapPosnFrntActForRowFirstForActrVentSideLe_read : Inport  Port=12
  AirFlapPosnFrntActForRowFirstForActrVentSideRi_AirFlapPosnFrntActForRowFirstForActrVentSideRi_read : Inport  Port=13
  AirFlapPosnFrntActForRowFirstForOsa_AirFlapPosnFrntActForRowFirstForOsa_read : Inport  Port=14
  AirFlapPosnFrntActForRowFirstForRecirc_AirFlapPosnFrntActForRowFirstForRecirc_read : Inport  Port=15
  AirFlapPosnFrntActForRowSecForActrFlr_AirFlapPosnFrntActForRowSecForActrFlr_read : Inport  Port=16
  AirFlapPosnFrntActForRowSecForActrTLe_AirFlapPosnFrntActForRowSecForActrTLe_read : Inport  Port=17
  AirFlapPosnFrntActForRowSecForActrTRi_AirFlapPosnFrntActForRowSecForActrTRi_read : Inport  Port=18
  AirFlapPosnFrntActForRowSecForActrVent_AirFlapPosnFrntActForRowSecForActrVent_read : Inport  Port=19
  AmbTFildForClimaNgcc_AmbTFildForClima_read : Inport  Port=20
  ClimaCfgForActrModVrnt1_ClimaCfgForActrInd1_read : Inport  Port=21
  ClimaCfgForActrModVrnt1_ClimaCfgForActrModVrnt1_read : Inport  Port=22
  ClimaCfgForActrModVrnt1_ClimaCfgForActrModVrntCen_read : Inport  Port=23
  ClimaCtrlActvNgcc_ClimaCtrlActv_read : Inport  Port=24
  HvacCtrlrFanReqFrnt_HvacCtrlrFanReqFrnt_read : Inport  Port=25
  HvacCtrlrReqFrntForOsaAndRecirc_HvacCtrlrReqFrntForOsaAndRecirc_read : Inport  Port=26
  HvacCtrlrReqFrntForRowFirstForActrDefrst_HvacCtrlrReqFrntForRowFirstForActrDefrst_read : Inport  Port=27
  HvacCtrlrReqFrntForRowFirstForActrFlr_HvacCtrlrReqFrntForRowFirstForActrFlr_read : Inport  Port=28
  HvacCtrlrReqFrntForRowFirstForActrFlr_HvacCtrlrReqFrntForRowFirstForActrFlrLe_read : Inport  Port=29
  HvacCtrlrReqFrntForRowFirstForActrFlr_HvacCtrlrReqFrntForRowFirstForActrFlrRi_read : Inport  Port=30
  HvacCtrlrReqFrntForRowFirstForActrMod_HvacCtrlrReqFrntForRowFirstForActrMod_read : Inport  Port=31
  HvacCtrlrReqFrntForRowFirstForActrOsa_HvacCtrlrReqFrntForRowFirstForActrOsa_read : Inport  Port=32
  HvacCtrlrReqFrntForRowFirstForActrRecirc_HvacCtrlrReqFrntForRowFirstForActrRecirc_read : Inport  Port=33
  HvacCtrlrReqFrntForRowFirstForActrTLe_HvacCtrlrReqFrntForRowFirstForActrTLe_read : Inport  Port=34
  HvacCtrlrReqFrntForRowFirstForActrTRi_HvacCtrlrReqFrntForRowFirstForActrTRi_read : Inport  Port=35
  HvacCtrlrReqFrntForRowFirstForActrVent_HvacCtrlrReqFrntForRowFirstForActrVent_read : Inport  Port=36
  HvacCtrlrReqFrntForRowFirstForActrVent_HvacCtrlrReqFrntForRowFirstForActrVentLe_read : Inport  Port=37
  HvacCtrlrReqFrntForRowFirstForActrVent_HvacCtrlrReqFrntForRowFirstForActrVentRi_read : Inport  Port=38
  HvacCtrlrReqFrntForRowFirstForActrVent_HvacCtrlrReqFrntForRowFirstForActrVentSideLe_read : Inport  Port=39
  HvacCtrlrReqFrntForRowFirstForActrVent_HvacCtrlrReqFrntForRowFirstForActrVentSideRi_read : Inport  Port=40
  HvacCtrlrReqFrntForRowFirstLeForActrMod_HvacCtrlrReqFrntForRowFirstLeForActrMod_read : Inport  Port=41
  HvacCtrlrReqFrntForRowSecForActrFlr_HvacCtrlrReqFrntForRowSecForActrFlr_read : Inport  Port=42
  HvacCtrlrReqFrntForRowSecForActrFlr_HvacCtrlrReqFrntForRowSecForActrFlrLe_read : Inport  Port=43
  HvacCtrlrReqFrntForRowSecForActrFlr_HvacCtrlrReqFrntForRowSecForActrFlrRi_read : Inport  Port=44
  HvacCtrlrReqFrntForRowSecForActrModLe_HvacCtrlrReqFrntForRowSecForActrModLe_read : Inport  Port=45
  HvacCtrlrReqFrntForRowSecForActrModRi_HvacCtrlrReqFrntForRowSecForActrModRi_read : Inport  Port=46
  HvacCtrlrReqFrntForRowSecForActrTLe_HvacCtrlrReqFrntForRowSecForActrTLe_read : Inport  Port=47
  HvacCtrlrReqFrntForRowSecForActrTRi_HvacCtrlrReqFrntForRowSecForActrTRi_read : Inport  Port=48
  HvacCtrlrReqFrntForRowSecForActrVent_HvacCtrlrReqFrntForRowSecForActrVent_read : Inport  Port=49
  ManModDurgFanOffActv_ManModDurgFanOffActv_read : Inport  Port=50
  MemReadForHvacActrPosnReqFrnt_MemReadForHvacActrPosnReqFrnt_read : Inport  Port=51
  MemReqStsForHvac_MemReqStsForHvac_read : Inport  Port=52
  VehSpdLgtForClima_VehSpdLgtForClima_read : Inport  Port=53
  ClimaAtRowSecActvNgcc_ClimaAtRowSecActv_read : Inport  Port=54
  ClimaAtRowSecActvNgcc_ClimaAtRowSecActvLe_read : Inport  Port=55
  ClimaAtRowSecActvNgcc_ClimaAtRowSecActvRi_read : Inport  Port=56
  ClimaCfgForClimaCtrlMan1_ClimaCfgForClimaCtrlMan1_read : Inport  Port=57
  ClimaCfgForHvacRePrsnt1_ClimaCfgForHvacRePrsnt1_read : Inport  Port=58
  function : TriggerPort  TriggerType=function-call  VariantControl=(inherit)  SampleTimeType=periodic  SampleTime=-1
  Ground_{1..24} (24 alike) : Ground
  Terminator_{1..58} (58 alike) : Terminator
  HmiDefrstReqForClima_HmiDefrstReqForClima_write : Outport
      settings: EnsureOutportIsVirtual=on
  HvacFildReqFrntForFan_HvacFildReqFrntForFan_write : Outport  Port=2
      settings: EnsureOutportIsVirtual=on
  HvacFildReqFrntForRowFirstForActrDefrst_HvacFildReqFrntForRowFirstForActrDefrst_write : Outport  Port=3
      settings: EnsureOutportIsVirtual=on
  HvacFildReqFrntForRowFirstForActrFlr_HvacFildReqFrntForRowFirstForActrFlr_write : Outport  Port=4
      settings: EnsureOutportIsVirtual=on
  HvacFildReqFrntForRowFirstForActrFlr_HvacFildReqFrntForRowFirstForActrFlrLe_write : Outport  Port=5
      settings: EnsureOutportIsVirtual=on
  HvacFildReqFrntForRowFirstForActrFlr_HvacFildReqFrntForRowFirstForActrFlrRi_write : Outport  Port=6
      settings: EnsureOutportIsVirtual=on
  HvacFildReqFrntForRowFirstForActrMod_HvacFildReqFrntForRowFirstForActrMod_write : Outport  Port=7
      settings: EnsureOutportIsVirtual=on
  HvacFildReqFrntForRowFirstForActrTLe_HvacFildReqFrntForRowFirstForActrTLe_write : Outport  Port=8
      settings: EnsureOutportIsVirtual=on
  HvacFildReqFrntForRowFirstForActrTRi_HvacFildReqFrntForRowFirstForActrTRi_write : Outport  Port=9
      settings: EnsureOutportIsVirtual=on
  HvacFildReqFrntForRowFirstForActrVent_HvacFildReqFrntForRowFirstForActrVent_write : Outport  Port=10
      settings: EnsureOutportIsVirtual=on
  HvacFildReqFrntForRowFirstForActrVent_HvacFildReqFrntForRowFirstForActrVentLe_write : Outport  Port=11
      settings: EnsureOutportIsVirtual=on
  HvacFildReqFrntForRowFirstForActrVent_HvacFildReqFrntForRowFirstForActrVentRi_write : Outport  Port=12
      settings: EnsureOutportIsVirtual=on
  HvacFildReqFrntForRowFirstForActrVent_HvacFildReqFrntForRowFirstForActrVentSideLe_write : Outport  Port=13
      settings: EnsureOutportIsVirtual=on
  HvacFildReqFrntForRowFirstForActrVent_HvacFildReqFrntForRowFirstForActrVentSideRi_write : Outport  Port=14
      settings: EnsureOutportIsVirtual=on
  HvacFildReqFrntForRowFirstForOsa_HvacFildReqFrntForRowFirstForOsa_write : Outport  Port=15
      settings: EnsureOutportIsVirtual=on
  HvacFildReqFrntForRowFirstForOsaAndRecirc_HvacFildReqFrntForRowFirstForOsaAndRecirc_write : Outport  Port=16
      settings: EnsureOutportIsVirtual=on
  HvacFildReqFrntForRowFirstForRecirc_HvacFildReqFrntForRowFirstForRecirc_write : Outport  Port=17
      settings: EnsureOutportIsVirtual=on
  HvacFildReqFrntForRowFirstLeForActrMod_HvacFildReqFrntForRowFirstLeForActrMod_write : Outport  Port=18
      settings: EnsureOutportIsVirtual=on
  HvacFildReqFrntForRowSecForActrFlr_HvacFildReqFrntForRowSecForActrFlr_write : Outport  Port=19
      settings: EnsureOutportIsVirtual=on
  HvacFildReqFrntForRowSecForActrModLe_HvacFildReqFrntForRowSecForActrModLe_write : Outport  Port=20
      settings: EnsureOutportIsVirtual=on
  HvacFildReqFrntForRowSecForActrModRi_HvacFildReqFrntForRowSecForActrModRi_write : Outport  Port=21
      settings: EnsureOutportIsVirtual=on
  HvacFildReqFrntForRowSecForActrTLe_HvacFildReqFrntForRowSecForActrTLe_write : Outport  Port=22
      settings: EnsureOutportIsVirtual=on
  HvacFildReqFrntForRowSecForActrTRi_HvacFildReqFrntForRowSecForActrTRi_write : Outport  Port=23
      settings: EnsureOutportIsVirtual=on
  HvacFildReqFrntForRowSecForActrVent_HvacFildReqFrntForRowSecForActrVent_write : Outport  Port=24
      settings: EnsureOutportIsVirtual=on
  wires:
  ActrPosnFrntActForOsaAndRecirc_ActrPosnFrntActForOsaAndRecirc_read/1 -> Terminator_1/1
  ActrPosnFrntActForRowFirstModDistbn_ActrPosnFrntActForRowFirstModDistbn_read/1 -> Terminator_2/1
  AirFlapPosnFrntActForRowFirstForActrDefrst_AirFlapPosnFrntActForRowFirstForActrDefrst_read/1 -> Terminator_3/1
  AirFlapPosnFrntActForRowFirstForActrFlr_AirFlapPosnFrntActForRowFirstForActrFlr_read/1 -> Terminator_4/1
  AirFlapPosnFrntActForRowFirstForActrFlrLe_AirFlapPosnFrntActForRowFirstForActrFlrLe_read/1 -> Terminator_5/1
  AirFlapPosnFrntActForRowFirstForActrFlrRi_AirFlapPosnFrntActForRowFirstForActrFlrRi_read/1 -> Terminator_6/1
  AirFlapPosnFrntActForRowFirstForActrTLe_AirFlapPosnFrntActForRowFirstForActrTLe_read/1 -> Terminator_7/1
  AirFlapPosnFrntActForRowFirstForActrTRi_AirFlapPosnFrntActForRowFirstForActrTRi_read/1 -> Terminator_8/1
  AirFlapPosnFrntActForRowFirstForActrVent_AirFlapPosnFrntActForRowFirstForActrVent_read/1 -> Terminator_9/1
  AirFlapPosnFrntActForRowFirstForActrVentLe_AirFlapPosnFrntActForRowFirstForActrVentLe_read/1 -> Terminator_10/1
  AirFlapPosnFrntActForRowFirstForActrVentRi_AirFlapPosnFrntActForRowFirstForActrVentRi_read/1 -> Terminator_11/1
  AirFlapPosnFrntActForRowFirstForActrVentSideLe_AirFlapPosnFrntActForRowFirstForActrVentSideLe_read/1 -> Terminator_12/1
  AirFlapPosnFrntActForRowFirstForActrVentSideRi_AirFlapPosnFrntActForRowFirstForActrVentSideRi_read/1 -> Terminator_13/1
  AirFlapPosnFrntActForRowFirstForOsa_AirFlapPosnFrntActForRowFirstForOsa_read/1 -> Terminator_14/1
  AirFlapPosnFrntActForRowFirstForRecirc_AirFlapPosnFrntActForRowFirstForRecirc_read/1 -> Terminator_15/1
  AirFlapPosnFrntActForRowSecForActrFlr_AirFlapPosnFrntActForRowSecForActrFlr_read/1 -> Terminator_16/1
  AirFlapPosnFrntActForRowSecForActrTLe_AirFlapPosnFrntActForRowSecForActrTLe_read/1 -> Terminator_17/1
  AirFlapPosnFrntActForRowSecForActrTRi_AirFlapPosnFrntActForRowSecForActrTRi_read/1 -> Terminator_18/1
  AirFlapPosnFrntActForRowSecForActrVent_AirFlapPosnFrntActForRowSecForActrVent_read/1 -> Terminator_19/1
  AmbTFildForClimaNgcc_AmbTFildForClima_read/1 -> Terminator_20/1
  ClimaCfgForActrModVrnt1_ClimaCfgForActrInd1_read/1 -> Terminator_21/1
  ClimaCfgForActrModVrnt1_ClimaCfgForActrModVrnt1_read/1 -> Terminator_22/1
  ClimaCfgForActrModVrnt1_ClimaCfgForActrModVrntCen_read/1 -> Terminator_23/1
  ClimaCtrlActvNgcc_ClimaCtrlActv_read/1 -> Terminator_24/1
  HvacCtrlrFanReqFrnt_HvacCtrlrFanReqFrnt_read/1 -> Terminator_25/1
  HvacCtrlrReqFrntForOsaAndRecirc_HvacCtrlrReqFrntForOsaAndRecirc_read/1 -> Terminator_26/1
  HvacCtrlrReqFrntForRowFirstForActrDefrst_HvacCtrlrReqFrntForRowFirstForActrDefrst_read/1 -> Terminator_27/1
  HvacCtrlrReqFrntForRowFirstForActrFlr_HvacCtrlrReqFrntForRowFirstForActrFlr_read/1 -> Terminator_28/1
  HvacCtrlrReqFrntForRowFirstForActrFlr_HvacCtrlrReqFrntForRowFirstForActrFlrLe_read/1 -> Terminator_29/1
  HvacCtrlrReqFrntForRowFirstForActrFlr_HvacCtrlrReqFrntForRowFirstForActrFlrRi_read/1 -> Terminator_30/1
  HvacCtrlrReqFrntForRowFirstForActrMod_HvacCtrlrReqFrntForRowFirstForActrMod_read/1 -> Terminator_31/1
  HvacCtrlrReqFrntForRowFirstForActrOsa_HvacCtrlrReqFrntForRowFirstForActrOsa_read/1 -> Terminator_32/1
  HvacCtrlrReqFrntForRowFirstForActrRecirc_HvacCtrlrReqFrntForRowFirstForActrRecirc_read/1 -> Terminator_33/1
  HvacCtrlrReqFrntForRowFirstForActrTLe_HvacCtrlrReqFrntForRowFirstForActrTLe_read/1 -> Terminator_34/1
  HvacCtrlrReqFrntForRowFirstForActrTRi_HvacCtrlrReqFrntForRowFirstForActrTRi_read/1 -> Terminator_35/1
  HvacCtrlrReqFrntForRowFirstForActrVent_HvacCtrlrReqFrntForRowFirstForActrVent_read/1 -> Terminator_36/1
  HvacCtrlrReqFrntForRowFirstForActrVent_HvacCtrlrReqFrntForRowFirstForActrVentLe_read/1 -> Terminator_37/1
  HvacCtrlrReqFrntForRowFirstForActrVent_HvacCtrlrReqFrntForRowFirstForActrVentRi_read/1 -> Terminator_38/1
  HvacCtrlrReqFrntForRowFirstForActrVent_HvacCtrlrReqFrntForRowFirstForActrVentSideLe_read/1 -> Terminator_39/1
  HvacCtrlrReqFrntForRowFirstForActrVent_HvacCtrlrReqFrntForRowFirstForActrVentSideRi_read/1 -> Terminator_40/1
  HvacCtrlrReqFrntForRowFirstLeForActrMod_HvacCtrlrReqFrntForRowFirstLeForActrMod_read/1 -> Terminator_41/1
  HvacCtrlrReqFrntForRowSecForActrFlr_HvacCtrlrReqFrntForRowSecForActrFlr_read/1 -> Terminator_42/1
  HvacCtrlrReqFrntForRowSecForActrFlr_HvacCtrlrReqFrntForRowSecForActrFlrLe_read/1 -> Terminator_43/1
  HvacCtrlrReqFrntForRowSecForActrFlr_HvacCtrlrReqFrntForRowSecForActrFlrRi_read/1 -> Terminator_44/1
  HvacCtrlrReqFrntForRowSecForActrModLe_HvacCtrlrReqFrntForRowSecForActrModLe_read/1 -> Terminator_45/1
  HvacCtrlrReqFrntForRowSecForActrModRi_HvacCtrlrReqFrntForRowSecForActrModRi_read/1 -> Terminator_46/1
  HvacCtrlrReqFrntForRowSecForActrTLe_HvacCtrlrReqFrntForRowSecForActrTLe_read/1 -> Terminator_47/1
  HvacCtrlrReqFrntForRowSecForActrTRi_HvacCtrlrReqFrntForRowSecForActrTRi_read/1 -> Terminator_48/1
  HvacCtrlrReqFrntForRowSecForActrVent_HvacCtrlrReqFrntForRowSecForActrVent_read/1 -> Terminator_49/1
  ManModDurgFanOffActv_ManModDurgFanOffActv_read/1 -> Terminator_50/1
  MemReadForHvacActrPosnReqFrnt_MemReadForHvacActrPosnReqFrnt_read/1 -> Terminator_51/1
  MemReqStsForHvac_MemReqStsForHvac_read/1 -> Terminator_52/1
  VehSpdLgtForClima_VehSpdLgtForClima_read/1 -> Terminator_53/1
  ClimaAtRowSecActvNgcc_ClimaAtRowSecActv_read/1 -> Terminator_54/1
  ClimaAtRowSecActvNgcc_ClimaAtRowSecActvLe_read/1 -> Terminator_55/1
  ClimaAtRowSecActvNgcc_ClimaAtRowSecActvRi_read/1 -> Terminator_56/1
  ClimaCfgForClimaCtrlMan1_ClimaCfgForClimaCtrlMan1_read/1 -> Terminator_57/1
  ClimaCfgForHvacRePrsnt1_ClimaCfgForHvacRePrsnt1_read/1 -> Terminator_58/1
  Ground_1/1 -> HmiDefrstReqForClima_HmiDefrstReqForClima_write/1
  Ground_2/1 -> HvacFildReqFrntForFan_HvacFildReqFrntForFan_write/1
  Ground_3/1 -> HvacFildReqFrntForRowFirstForActrDefrst_HvacFildReqFrntForRowFirstForActrDefrst_write/1
  Ground_4/1 -> HvacFildReqFrntForRowFirstForActrFlr_HvacFildReqFrntForRowFirstForActrFlr_write/1
  Ground_5/1 -> HvacFildReqFrntForRowFirstForActrFlr_HvacFildReqFrntForRowFirstForActrFlrLe_write/1
  Ground_6/1 -> HvacFildReqFrntForRowFirstForActrFlr_HvacFildReqFrntForRowFirstForActrFlrRi_write/1
  Ground_7/1 -> HvacFildReqFrntForRowFirstForActrMod_HvacFildReqFrntForRowFirstForActrMod_write/1
  Ground_8/1 -> HvacFildReqFrntForRowFirstForActrTLe_HvacFildReqFrntForRowFirstForActrTLe_write/1
  Ground_9/1 -> HvacFildReqFrntForRowFirstForActrTRi_HvacFildReqFrntForRowFirstForActrTRi_write/1
  Ground_10/1 -> HvacFildReqFrntForRowFirstForActrVent_HvacFildReqFrntForRowFirstForActrVent_write/1
  Ground_11/1 -> HvacFildReqFrntForRowFirstForActrVent_HvacFildReqFrntForRowFirstForActrVentLe_write/1
  Ground_12/1 -> HvacFildReqFrntForRowFirstForActrVent_HvacFildReqFrntForRowFirstForActrVentRi_write/1
  Ground_13/1 -> HvacFildReqFrntForRowFirstForActrVent_HvacFildReqFrntForRowFirstForActrVentSideLe_write/1
  Ground_14/1 -> HvacFildReqFrntForRowFirstForActrVent_HvacFildReqFrntForRowFirstForActrVentSideRi_write/1
  Ground_15/1 -> HvacFildReqFrntForRowFirstForOsa_HvacFildReqFrntForRowFirstForOsa_write/1
  Ground_16/1 -> HvacFildReqFrntForRowFirstForOsaAndRecirc_HvacFildReqFrntForRowFirstForOsaAndRecirc_write/1
  Ground_17/1 -> HvacFildReqFrntForRowFirstForRecirc_HvacFildReqFrntForRowFirstForRecirc_write/1
  Ground_18/1 -> HvacFildReqFrntForRowFirstLeForActrMod_HvacFildReqFrntForRowFirstLeForActrMod_write/1
  Ground_19/1 -> HvacFildReqFrntForRowSecForActrFlr_HvacFildReqFrntForRowSecForActrFlr_write/1
  Ground_20/1 -> HvacFildReqFrntForRowSecForActrModLe_HvacFildReqFrntForRowSecForActrModLe_write/1
  Ground_21/1 -> HvacFildReqFrntForRowSecForActrModRi_HvacFildReqFrntForRowSecForActrModRi_write/1
  Ground_22/1 -> HvacFildReqFrntForRowSecForActrTLe_HvacFildReqFrntForRowSecForActrTLe_write/1
  Ground_23/1 -> HvacFildReqFrntForRowSecForActrTRi_HvacFildReqFrntForRowSecForActrTRi_write/1
  Ground_24/1 -> HvacFildReqFrntForRowSecForActrVent_HvacFildReqFrntForRowSecForActrVent_write/1
